simulink model "Demo_TrafficFlow_cs"  (Simulink 7.6)
settings: Solver=ode5  SolverMode=SingleTasking  StartTime=0.0  StopTime=60  FixedStep=1/20  RelTol=1e-3
PreLoadFcn:
  | check_NUTS_defined
  | try
  |     evalin('base', 'NUTS_defined;');
  | catch
  |     warningMsg = sprintf('PreScan Warning: \nUsing this model requires that MATLAB is configured for PreScan. The model may not display correctly.\n\n ==> Please open MATLAB with the dedicated buttons provided with PreScan');
  |     disp(warningMsg)
  |     warndlg(warningMsg,'WindowStyle','Modal');
  | end
  | 
  | model_closed = 0;
  | assignin('base',['model_closed_' bdroot],false);
InitFcn:
  | PreScanExperimentInitFcn(bdroot)
StopFcn:
  | PreScanExperimentStopFcn(bdroot)
undefined symbols (not defined in the file or in its folder): Advised_Speed_L1, Advised_Speed_L2, Advised_Speed_L3, DiscIntNeverNeededParam, spare_10, spare_5, spare_6, spare_7, spare_8, spare_9, State_L2, Throughput_L1, Throughput_L2, Throughput_L3, Velocity_L1, Velocity_L2, Velocity_L3
libraries: simulink x58, PreScanSimulinkLibrary x40, simulink_extras x32, PreScanUsefulBlocks x22, simulink_need_slupdate x3
(part 3 of 20)

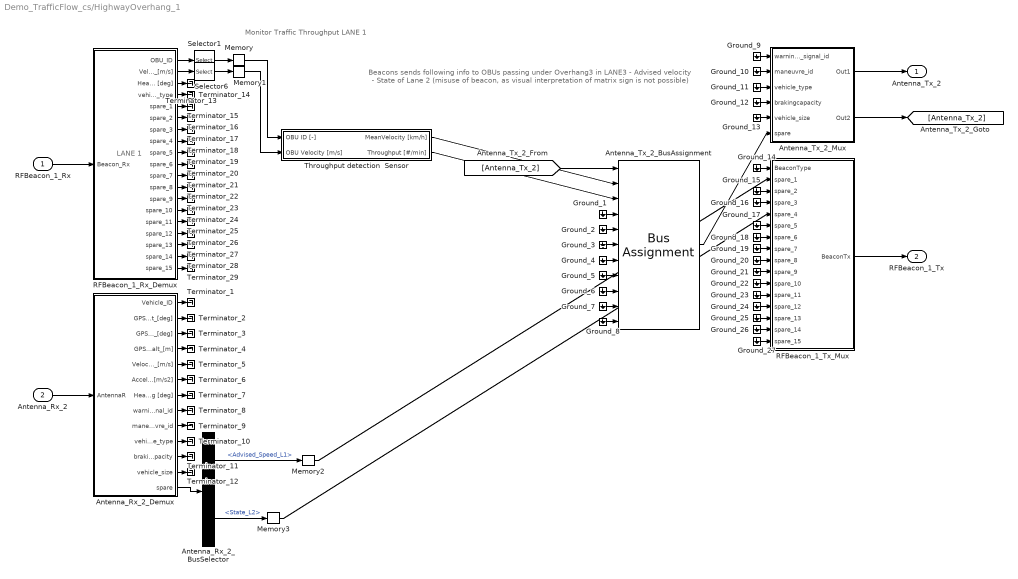
== system Demo_TrafficFlow_cs/HighwayOverhang_1 ==
  note: LANE 1
  note: Monitor Traffic Throughput LANE 1
  note: Beacons sends following info to OBUs
      | passing under Overhang3 in LANE3
      | - Advised velocity
      | - State of Lane 2 (misuse of beacon, as visual
      |    interpretation of matrix sign is not possible)
  RFBeacon_1_Rx : Inport  Tag=BeaconRxInport
  Antenna_Rx_2 : Inport  Tag=AntennaRInport  Port=2
  Antenna_Rx_2_BusSelector : BusSelector  Tag=BusAntennaR  OutputSignals=Advised_Speed_L1,State_L2
  Antenna_Rx_2_Demux : Subsystem  Tag=DemuxAntennaR  mask(PreScan Demux Block:){Channel=1}
      settings: DeleteFcn=PreScanBlockDeleteFcn;
      mask Display:
      | fprintf('Channel: %d',Channel)
      | port_label('output', 1, 'Vehicle_ID')
      | port_label('output', 2, 'GPS Pos Lat [deg]')
      | port_label('output', 3, 'GPS Pos Long [deg]')
      | port_label('output', 4, 'GPS Pos Alt [m]')
      | port_label('output', 5, 'Velocity [m/s]')
      | port_label('output', 6, 'Acceleration [m/s2]')
      | port_label('output', 7, 'Heading [deg]')
      | port_label('output', 8, 'Warning signal ID')
      | port_label('output', 9, 'Maneuvre ID')
      | port_label('output', 10, 'Vehicle Type')
      | port_label('output', 11, 'Braking Capacity')
      | port_label('output', 12, 'Vehicle Size')
      | port_label('output', 13, 'Spare')
  Antenna_Tx_2_BusAssignment : BusAssignment  Tag=BusAntennaT  AssignedSignals=Velocity_L1,Throughput_L1,Advised_Speed_L1,State_L2,spare_5,spare_6,spare_7,spare_8,spare_9,spare_10
  Antenna_Tx_2_Mux : Subsystem  Tag=MuxAntennaT  mask(PreScan Mux Block:){Channel=1, TimeStep=1/20}
      mask prompts: TimeStep "Time Step [s]"
      settings: DeleteFcn=PreScanBlockDeleteFcn;
      mask Display:
      | fprintf('Channel: %d',Channel)
      | port_label('input', 1, 'Warning signal ID')
      | port_label('input', 2, 'Maneuvre ID')
      | port_label('input', 3, 'Vehicle Type')
      | port_label('input', 4, 'Braking Capacity')
      | port_label('input', 5, 'Vehicle Size')
      | port_label('input', 6, 'spare')
  Ground_{1..27} (27 alike) : Ground
  Memory{,1..3} (4 alike) : Memory
  RFBeacon_1_Rx_Demux : Subsystem  Tag=DemuxAntennaR  mask(PreScan Demux Block:){Technology='RF'}
      mask init:
      | IconTxt = char('','','','','Technology:            ',['{\it',Technology,'}']);
      settings: DeleteFcn=PreScanBlockDeleteFcn;
      mask Display:
      | disp(IconTxt,'texmode','on')
      | port_label('output', 1, 'OBU ID [-]')
      | port_label('output', 2, 'OBU Velocity [m/s]')
      | port_label('output', 3, 'OBU Heading [deg]')
      | port_label('output', 4, 'Vehicle Type[-]')
      | port_label('output', 5, 'spare_1')
      | port_label('output', 6, 'spare_2')
      | port_label('output', 7, 'spare_3')
      | port_label('output', 8, 'spare_4')
      | port_label('output', 9, 'spare_5')
      | port_label('output', 10, 'spare_6')
      | port_label('output', 11, 'spare_7')
      | port_label('output', 12, 'spare_8')
      | port_label('output', 13, 'spare_9')
      | port_label('output', 14, 'spare_10')
      | port_label('output', 15, 'spare_11')
      | port_label('output', 16, 'spare_12')
      | port_label('output', 17, 'spare_13')
      | port_label('output', 18, 'spare_14')
      | port_label('output', 19, 'spare_15')
  RFBeacon_1_Tx_Mux : Subsystem  Tag=MuxBeaconTx  mask(PreScan Mux Block:){Technology='RF'}
      mask init:
      | IconTxt = char('','','','              Technology:',['{\it                ',Technology,'}']);
      settings: DeleteFcn=PreScanBlockDeleteFcn;
      mask Display:
      | disp(IconTxt,'texmode','on')
      | port_label('input', 1, 'Beacon Type [-]')
      | port_label('input', 2, 'Advised_Velocity')
      | port_label('input', 3, 'CarID_Lane1')
      | port_label('input', 4, 'CarID_Lane3')
      | port_label('input', 5, 'State_Lane2')
      | port_label('input', 6, 'spare_5')
      | port_label('input', 7, 'spare_6')
      | port_label('input', 8, 'spare_7')
      | port_label('input', 9, 'spare_8')
      | port_label('input', 10, 'spare_9')
      | port_label('input', 11, 'spare_10')
      | port_label('input', 12, 'spare_11')
      | port_label('input', 13, 'spare_12')
      | port_label('input', 14, 'spare_13')
      | port_label('input', 15, 'spare_14')
      | port_label('input', 16, 'spare_15')
  Selector1 : Selector  InputPortWidth=5  IndexOptions=Index vector (dialog)  Indices=[1]  OutputSizes=1
  Selector6 : Selector  InputPortWidth=5  IndexOptions=Index vector (dialog)  Indices=[1]  OutputSizes=1
  Terminator_{1..29} (29 alike) : Terminator
  Throughput detection  Sensor : Subsystem
  Antenna_Tx_2 : Outport  Tag=AntennaTOutport
  RFBeacon_1_Tx : Outport  Tag=AntennaTOutport  Port=2
  wires:
  Selector6/1 -> Memory1/1
  Selector1/1 -> Memory/1
  Memory/1 -> Throughput detection  Sensor/1(OBU ID [-])
  Memory1/1 -> Throughput detection  Sensor/2(OBU Velocity [m/s])
  RFBeacon_1_Rx/1 -> RFBeacon_1_Rx_Demux/1(Beacon_Rx)
  Antenna_Rx_2_Demux/13(spare) -> Antenna_Rx_2_BusSelector/1
  Antenna_Rx_2/1 -> Antenna_Rx_2_Demux/1(AntennaR)
  Antenna_Tx_2_Mux/2(Out2) -> [Antenna_Tx_2]
  [Antenna_Tx_2] -> Antenna_Tx_2_BusAssignment/1
  Antenna_Tx_2_BusAssignment/1 -> Antenna_Tx_2_Mux/6(spare)
  Antenna_Tx_2_Mux/1(Out1) -> Antenna_Tx_2/1
  RFBeacon_1_Tx_Mux/1(BeaconTx) -> RFBeacon_1_Tx/1
  Memory3/1 -> RFBeacon_1_Tx_Mux/5(spare_4)
  RFBeacon_1_Rx_Demux/2(Velocity_[m/s]) -> Selector6/1
  RFBeacon_1_Rx_Demux/1(OBU_ID) -> Selector1/1
  Throughput detection  Sensor/2(Throughput [#/min]) -> Antenna_Tx_2_BusAssignment/3
  Throughput detection  Sensor/1(MeanVelocity [km/h]) -> Antenna_Tx_2_BusAssignment/2
  Memory2/1 -> RFBeacon_1_Tx_Mux/2(spare_1)
  Antenna_Rx_2_BusSelector/1 -> Memory2/1  "<Advised_Speed_L1>"
  Antenna_Rx_2_BusSelector/2 -> Memory3/1  "<State_L2>"
  Antenna_Rx_2_Demux/1(Vehicle_ID) -> Terminator_1/1
  Antenna_Rx_2_Demux/2(GPS_pos_lat_[deg]) -> Terminator_2/1
  Antenna_Rx_2_Demux/3(GPS_pos_long_[deg]) -> Terminator_3/1
  Antenna_Rx_2_Demux/4(GPS_pos_alt_[m]) -> Terminator_4/1
  Antenna_Rx_2_Demux/5(Velocity_[m/s]) -> Terminator_5/1
  Antenna_Rx_2_Demux/6(Acceleration_[m/s2]) -> Terminator_6/1
  Antenna_Rx_2_Demux/7(Heading [deg]) -> Terminator_7/1
  Antenna_Rx_2_Demux/8(warning_signal_id) -> Terminator_8/1
  Antenna_Rx_2_Demux/9(maneuvre_id) -> Terminator_9/1
  Antenna_Rx_2_Demux/10(vehicle_type) -> Terminator_10/1
  Antenna_Rx_2_Demux/11(brakingcapacity) -> Terminator_11/1
  Antenna_Rx_2_Demux/12(vehicle_size) -> Terminator_12/1
  Ground_1/1 -> Antenna_Tx_2_BusAssignment/4
  Ground_2/1 -> Antenna_Tx_2_BusAssignment/5
  Ground_3/1 -> Antenna_Tx_2_BusAssignment/6
  Ground_4/1 -> Antenna_Tx_2_BusAssignment/7
  Ground_5/1 -> Antenna_Tx_2_BusAssignment/8
  Ground_6/1 -> Antenna_Tx_2_BusAssignment/9
  Ground_7/1 -> Antenna_Tx_2_BusAssignment/10
  Ground_8/1 -> Antenna_Tx_2_BusAssignment/11
  Ground_9/1 -> Antenna_Tx_2_Mux/1(warning_signal_id)
  Ground_10/1 -> Antenna_Tx_2_Mux/2(maneuvre_id)
  Ground_11/1 -> Antenna_Tx_2_Mux/3(vehicle_type)
  Ground_12/1 -> Antenna_Tx_2_Mux/4(brakingcapacity)
  Ground_13/1 -> Antenna_Tx_2_Mux/5(vehicle_size)
  RFBeacon_1_Rx_Demux/3(Heading [deg]) -> Terminator_13/1
  RFBeacon_1_Rx_Demux/4(vehicle_type) -> Terminator_14/1
  RFBeacon_1_Rx_Demux/5(spare_1) -> Terminator_15/1
  RFBeacon_1_Rx_Demux/6(spare_2) -> Terminator_16/1
  RFBeacon_1_Rx_Demux/7(spare_3) -> Terminator_17/1
  RFBeacon_1_Rx_Demux/8(spare_4) -> Terminator_18/1
  RFBeacon_1_Rx_Demux/9(spare_5) -> Terminator_19/1
  RFBeacon_1_Rx_Demux/10(spare_6) -> Terminator_20/1
  RFBeacon_1_Rx_Demux/11(spare_7) -> Terminator_21/1
  RFBeacon_1_Rx_Demux/12(spare_8) -> Terminator_22/1
  RFBeacon_1_Rx_Demux/13(spare_9) -> Terminator_23/1
  RFBeacon_1_Rx_Demux/14(spare_10) -> Terminator_24/1
  RFBeacon_1_Rx_Demux/15(spare_11) -> Terminator_25/1
  RFBeacon_1_Rx_Demux/16(spare_12) -> Terminator_26/1
  RFBeacon_1_Rx_Demux/17(spare_13) -> Terminator_27/1
  RFBeacon_1_Rx_Demux/18(spare_14) -> Terminator_28/1
  RFBeacon_1_Rx_Demux/19(spare_15) -> Terminator_29/1
  Ground_14/1 -> RFBeacon_1_Tx_Mux/1(BeaconType)
  Ground_15/1 -> RFBeacon_1_Tx_Mux/3(spare_2)
  Ground_16/1 -> RFBeacon_1_Tx_Mux/4(spare_3)
  Ground_17/1 -> RFBeacon_1_Tx_Mux/6(spare_5)
  Ground_18/1 -> RFBeacon_1_Tx_Mux/7(spare_6)
  Ground_19/1 -> RFBeacon_1_Tx_Mux/8(spare_7)
  Ground_20/1 -> RFBeacon_1_Tx_Mux/9(spare_8)
  Ground_21/1 -> RFBeacon_1_Tx_Mux/10(spare_9)
  Ground_22/1 -> RFBeacon_1_Tx_Mux/11(spare_10)
  Ground_23/1 -> RFBeacon_1_Tx_Mux/12(spare_11)
  Ground_24/1 -> RFBeacon_1_Tx_Mux/13(spare_12)
  Ground_25/1 -> RFBeacon_1_Tx_Mux/14(spare_13)
  Ground_26/1 -> RFBeacon_1_Tx_Mux/15(spare_14)
  Ground_27/1 -> RFBeacon_1_Tx_Mux/16(spare_15)

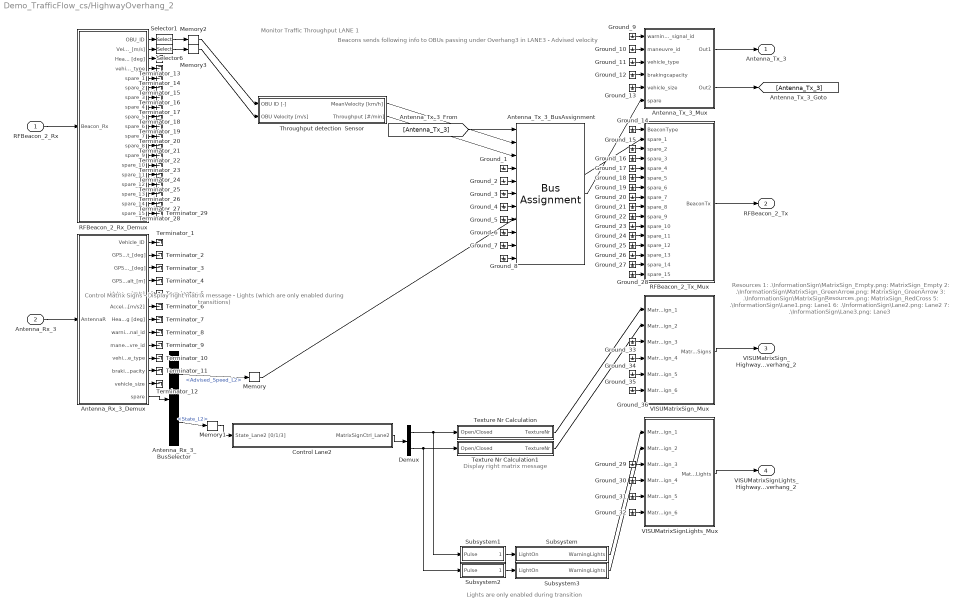
== system Demo_TrafficFlow_cs/HighwayOverhang_2 ==
  note: Lights are only enabled during transition
  note: Display right matrix message
  note: Monitor Traffic Throughput LANE 1
  note: Beacons sends following info to OBUs
      | passing under Overhang3 in LANE3
      | - Advised velocity
  note: Control Matrix Signs
      | - Display right matrix message
      | - Lights (which are only enabled during transitions)
  note: Resources
      |
      | 1: .\InformationSign\MatrixSign_Empty.png: MatrixSign_Empty
      | 2: .\InformationSign\MatrixSign_GreenArrow.png: MatrixSign_GreenArrow
      | 3: .\InformationSign\MatrixSign_RedCross.png: MatrixSign_RedCross
      | 5: .\InformationSign\Lane1.png: Lane1
      | 6: .\InformationSign\Lane2.png: Lane2
      | 7: .\InformationSign\Lane3.png: Lane3
  note: Resources
  RFBeacon_2_Rx : Inport  Tag=BeaconRxInport
  Antenna_Rx_3 : Inport  Tag=AntennaRInport  Port=2
  Antenna_Rx_3_BusSelector : BusSelector  Tag=BusAntennaR  OutputSignals=Advised_Speed_L2,State_L2
  Antenna_Rx_3_Demux : Subsystem  Tag=DemuxAntennaR  mask(PreScan Demux Block:){Channel=2}
      settings: DeleteFcn=PreScanBlockDeleteFcn;
      mask Display:
      | fprintf('Channel: %d',Channel)
      | port_label('output', 1, 'Vehicle_ID')
      | port_label('output', 2, 'GPS Pos Lat [deg]')
      | port_label('output', 3, 'GPS Pos Long [deg]')
      | port_label('output', 4, 'GPS Pos Alt [m]')
      | port_label('output', 5, 'Velocity [m/s]')
      | port_label('output', 6, 'Acceleration [m/s2]')
      | port_label('output', 7, 'Heading [deg]')
      | port_label('output', 8, 'Warning signal ID')
      | port_label('output', 9, 'Maneuvre ID')
      | port_label('output', 10, 'Vehicle Type')
      | port_label('output', 11, 'Braking Capacity')
      | port_label('output', 12, 'Vehicle Size')
      | port_label('output', 13, 'Spare')
  Antenna_Tx_3_BusAssignment : BusAssignment  Tag=BusAntennaT  AssignedSignals=Velocity_L2,Throughput_L2,Advised_Speed_L2,State_L2,spare_5,spare_6,spare_7,spare_8,spare_9,spare_10
  Antenna_Tx_3_Mux : Subsystem  Tag=MuxAntennaT  mask(PreScan Mux Block:){Channel=2, TimeStep=1/20}
      mask prompts: TimeStep "Time Step [s]"
      settings: DeleteFcn=PreScanBlockDeleteFcn;
      mask Display:
      | fprintf('Channel: %d',Channel)
      | port_label('input', 1, 'Warning signal ID')
      | port_label('input', 2, 'Maneuvre ID')
      | port_label('input', 3, 'Vehicle Type')
      | port_label('input', 4, 'Braking Capacity')
      | port_label('input', 5, 'Vehicle Size')
      | port_label('input', 6, 'spare')
  Control Lane2 : Subsystem
  Demux : Demux  Outputs=2
  Ground_{1..36} (36 alike) : Ground
  Memory{,1..3} (4 alike) : Memory
  RFBeacon_2_Rx_Demux : Subsystem  Tag=DemuxAntennaR  mask(PreScan Demux Block:){Technology='RF'}
      mask init:
      | IconTxt = char('','','','','Technology:            ',['{\it',Technology,'}']);
      settings: DeleteFcn=PreScanBlockDeleteFcn;
      mask Display:
      | disp(IconTxt,'texmode','on')
      | port_label('output', 1, 'OBU ID [-]')
      | port_label('output', 2, 'OBU Velocity [m/s]')
      | port_label('output', 3, 'OBU Heading [deg]')
      | port_label('output', 4, 'Vehicle Type[-]')
      | port_label('output', 5, 'spare_1')
      | port_label('output', 6, 'spare_2')
      | port_label('output', 7, 'spare_3')
      | port_label('output', 8, 'spare_4')
      | port_label('output', 9, 'spare_5')
      | port_label('output', 10, 'spare_6')
      | port_label('output', 11, 'spare_7')
      | port_label('output', 12, 'spare_8')
      | port_label('output', 13, 'spare_9')
      | port_label('output', 14, 'spare_10')
      | port_label('output', 15, 'spare_11')
      | port_label('output', 16, 'spare_12')
      | port_label('output', 17, 'spare_13')
      | port_label('output', 18, 'spare_14')
      | port_label('output', 19, 'spare_15')
  RFBeacon_2_Tx_Mux : Subsystem  Tag=MuxBeaconTx  mask(PreScan Mux Block:){Technology='RF'}
      mask init:
      | IconTxt = char('','','','              Technology:',['{\it                ',Technology,'}']);
      settings: DeleteFcn=PreScanBlockDeleteFcn;
      mask Display:
      | disp(IconTxt,'texmode','on')
      | port_label('input', 1, 'Beacon Type [-]')
      | port_label('input', 2, 'Advised_Velocity')
      | port_label('input', 3, 'CarID_Lane1')
      | port_label('input', 4, 'CarID_Lane3')
      | port_label('input', 5, 'State_Lane2')
      | port_label('input', 6, 'spare_5')
      | port_label('input', 7, 'spare_6')
      | port_label('input', 8, 'spare_7')
      | port_label('input', 9, 'spare_8')
      | port_label('input', 10, 'spare_9')
      | port_label('input', 11, 'spare_10')
      | port_label('input', 12, 'spare_11')
      | port_label('input', 13, 'spare_12')
      | port_label('input', 14, 'spare_13')
      | port_label('input', 15, 'spare_14')
      | port_label('input', 16, 'spare_15')
  Selector1 : Selector  InputPortWidth=5  IndexOptions=Index vector (dialog)  Indices=[1]  OutputSizes=1
  Selector6 : Selector  InputPortWidth=5  IndexOptions=Index vector (dialog)  Indices=[1]  OutputSizes=1
  Subsystem : Subsystem
  Subsystem1 : Subsystem  mask{t_light_on=10}
      mask prompts: t_light_on "Set duration of warning lights activation time"
  Subsystem2 : Subsystem  mask{t_light_on=3}
      mask prompts: t_light_on "Set duration of warning lights activation time"
  Subsystem3 : Subsystem
  Terminator_{1..29} (29 alike) : Terminator
  Texture Nr Calculation : Subsystem
  Texture Nr Calculation1 : Subsystem
  Throughput detection  Sensor : Subsystem
  VISUMatrixSignLights_Mux : Subsystem  Tag=MuxMatrixSignLights
      settings: DeleteFcn=PreScanBlockDeleteFcn;
      mask Type: PreScan Mux Block:
  VISUMatrixSign_Mux : Subsystem  Tag=MuxMatrixSign
      settings: DeleteFcn=PreScanBlockDeleteFcn;
      mask Type: PreScan Mux Block:
  Antenna_Tx_3 : Outport  Tag=AntennaTOutport
  RFBeacon_2_Tx : Outport  Tag=AntennaTOutport  Port=2
  VISUMatrixSign_HighwayOverhang_2 : Outport  Tag=VisuMatrixSignOutport  Port=3  SampleTime=1/20
  VISUMatrixSignLights_HighwayOverhang_2 : Outport  Tag=VisuMatrixSignLightsOutport  Port=4  SampleTime=1/20
  wires:
  Demux/1 -> Texture Nr Calculation/1(Open/Closed), Subsystem1/1(Pulse)
  Demux/2 -> Texture Nr Calculation1/1(Open/Closed), Subsystem2/1(Pulse)
  Subsystem2/1(LightOn) -> Subsystem3/1(LightOn)
  Subsystem1/1(LightOn) -> Subsystem/1(LightOn)
  Selector1/1 -> Memory2/1
  Selector6/1 -> Memory3/1
  Control Lane2/1(MatrixSignCtrl_Lane2) -> Demux/1
  Memory1/1 -> Control Lane2/1(State_Lane2 [0/1/3])
  Memory2/1 -> Throughput detection  Sensor/1(OBU ID [-])
  Memory3/1 -> Throughput detection  Sensor/2(OBU Velocity [m/s])
  RFBeacon_2_Rx/1 -> RFBeacon_2_Rx_Demux/1(Beacon_Rx)
  Antenna_Rx_3_Demux/13(spare) -> Antenna_Rx_3_BusSelector/1
  Antenna_Rx_3/1 -> Antenna_Rx_3_Demux/1(AntennaR)
  Antenna_Tx_3_Mux/2(Out2) -> [Antenna_Tx_3]
  [Antenna_Tx_3] -> Antenna_Tx_3_BusAssignment/1
  Antenna_Tx_3_BusAssignment/1 -> Antenna_Tx_3_Mux/6(spare)
  Antenna_Tx_3_Mux/1(Out1) -> Antenna_Tx_3/1
  RFBeacon_2_Tx_Mux/1(BeaconTx) -> RFBeacon_2_Tx/1
  VISUMatrixSign_Mux/1(MatrixSigns) -> VISUMatrixSign_HighwayOverhang_2/1
  VISUMatrixSignLights_Mux/1(MatrixSignLights) -> VISUMatrixSignLights_HighwayOverhang_2/1
  RFBeacon_2_Rx_Demux/1(OBU_ID) -> Selector1/1
  RFBeacon_2_Rx_Demux/2(Velocity_[m/s]) -> Selector6/1
  Subsystem/1(WarningLights) -> VISUMatrixSignLights_Mux/1(MatrixSign_1)
  Subsystem3/1(WarningLights) -> VISUMatrixSignLights_Mux/2(MatrixSign_2)
  Texture Nr Calculation/1(TextureNr) -> VISUMatrixSign_Mux/1(MatrixSign_1)
  Texture Nr Calculation1/1(TextureNr) -> VISUMatrixSign_Mux/2(MatrixSign_2)
  Memory/1 -> RFBeacon_2_Tx_Mux/2(spare_1)
  Throughput detection  Sensor/1(MeanVelocity [km/h]) -> Antenna_Tx_3_BusAssignment/2
  Throughput detection  Sensor/2(Throughput [#/min]) -> Antenna_Tx_3_BusAssignment/3
  Antenna_Rx_3_BusSelector/1 -> Memory/1  "<Advised_Speed_L2>"
  Antenna_Rx_3_BusSelector/2 -> Memory1/1  "<State_L2>"
  Antenna_Rx_3_Demux/1(Vehicle_ID) -> Terminator_1/1
  Antenna_Rx_3_Demux/2(GPS_pos_lat_[deg]) -> Terminator_2/1
  Antenna_Rx_3_Demux/3(GPS_pos_long_[deg]) -> Terminator_3/1
  Antenna_Rx_3_Demux/4(GPS_pos_alt_[m]) -> Terminator_4/1
  Antenna_Rx_3_Demux/5(Velocity_[m/s]) -> Terminator_5/1
  Antenna_Rx_3_Demux/6(Acceleration_[m/s2]) -> Terminator_6/1
  Antenna_Rx_3_Demux/7(Heading [deg]) -> Terminator_7/1
  Antenna_Rx_3_Demux/8(warning_signal_id) -> Terminator_8/1
  Antenna_Rx_3_Demux/9(maneuvre_id) -> Terminator_9/1
  Antenna_Rx_3_Demux/10(vehicle_type) -> Terminator_10/1
  Antenna_Rx_3_Demux/11(brakingcapacity) -> Terminator_11/1
  Antenna_Rx_3_Demux/12(vehicle_size) -> Terminator_12/1
  Ground_1/1 -> Antenna_Tx_3_BusAssignment/4
  Ground_2/1 -> Antenna_Tx_3_BusAssignment/5
  Ground_3/1 -> Antenna_Tx_3_BusAssignment/6
  Ground_4/1 -> Antenna_Tx_3_BusAssignment/7
  Ground_5/1 -> Antenna_Tx_3_BusAssignment/8
  Ground_6/1 -> Antenna_Tx_3_BusAssignment/9
  Ground_7/1 -> Antenna_Tx_3_BusAssignment/10
  Ground_8/1 -> Antenna_Tx_3_BusAssignment/11
  Ground_9/1 -> Antenna_Tx_3_Mux/1(warning_signal_id)
  Ground_10/1 -> Antenna_Tx_3_Mux/2(maneuvre_id)
  Ground_11/1 -> Antenna_Tx_3_Mux/3(vehicle_type)
  Ground_12/1 -> Antenna_Tx_3_Mux/4(brakingcapacity)
  Ground_13/1 -> Antenna_Tx_3_Mux/5(vehicle_size)
  RFBeacon_2_Rx_Demux/3(Heading [deg]) -> Terminator_13/1
  RFBeacon_2_Rx_Demux/4(vehicle_type) -> Terminator_14/1
  RFBeacon_2_Rx_Demux/5(spare_1) -> Terminator_15/1
  RFBeacon_2_Rx_Demux/6(spare_2) -> Terminator_16/1
  RFBeacon_2_Rx_Demux/7(spare_3) -> Terminator_17/1
  RFBeacon_2_Rx_Demux/8(spare_4) -> Terminator_18/1
  RFBeacon_2_Rx_Demux/9(spare_5) -> Terminator_19/1
  RFBeacon_2_Rx_Demux/10(spare_6) -> Terminator_20/1
  RFBeacon_2_Rx_Demux/11(spare_7) -> Terminator_21/1
  RFBeacon_2_Rx_Demux/12(spare_8) -> Terminator_22/1
  RFBeacon_2_Rx_Demux/13(spare_9) -> Terminator_23/1
  RFBeacon_2_Rx_Demux/14(spare_10) -> Terminator_24/1
  RFBeacon_2_Rx_Demux/15(spare_11) -> Terminator_25/1
  RFBeacon_2_Rx_Demux/16(spare_12) -> Terminator_26/1
  RFBeacon_2_Rx_Demux/17(spare_13) -> Terminator_27/1
  RFBeacon_2_Rx_Demux/18(spare_14) -> Terminator_28/1
  RFBeacon_2_Rx_Demux/19(spare_15) -> Terminator_29/1
  Ground_14/1 -> RFBeacon_2_Tx_Mux/1(BeaconType)
  Ground_15/1 -> RFBeacon_2_Tx_Mux/3(spare_2)
  Ground_16/1 -> RFBeacon_2_Tx_Mux/4(spare_3)
  Ground_17/1 -> RFBeacon_2_Tx_Mux/5(spare_4)
  Ground_18/1 -> RFBeacon_2_Tx_Mux/6(spare_5)
  Ground_19/1 -> RFBeacon_2_Tx_Mux/7(spare_6)
  Ground_20/1 -> RFBeacon_2_Tx_Mux/8(spare_7)
  Ground_21/1 -> RFBeacon_2_Tx_Mux/9(spare_8)
  Ground_22/1 -> RFBeacon_2_Tx_Mux/10(spare_9)
  Ground_23/1 -> RFBeacon_2_Tx_Mux/11(spare_10)
  Ground_24/1 -> RFBeacon_2_Tx_Mux/12(spare_11)
  Ground_25/1 -> RFBeacon_2_Tx_Mux/13(spare_12)
  Ground_26/1 -> RFBeacon_2_Tx_Mux/14(spare_13)
  Ground_27/1 -> RFBeacon_2_Tx_Mux/15(spare_14)
  Ground_28/1 -> RFBeacon_2_Tx_Mux/16(spare_15)
  Ground_29/1 -> VISUMatrixSignLights_Mux/3(MatrixSign_3)
  Ground_30/1 -> VISUMatrixSignLights_Mux/4(MatrixSign_4)
  Ground_31/1 -> VISUMatrixSignLights_Mux/5(MatrixSign_5)
  Ground_32/1 -> VISUMatrixSignLights_Mux/6(MatrixSign_6)
  Ground_33/1 -> VISUMatrixSign_Mux/3(MatrixSign_3)
  Ground_34/1 -> VISUMatrixSign_Mux/4(MatrixSign_4)
  Ground_35/1 -> VISUMatrixSign_Mux/5(MatrixSign_5)
  Ground_36/1 -> VISUMatrixSign_Mux/6(MatrixSign_6)

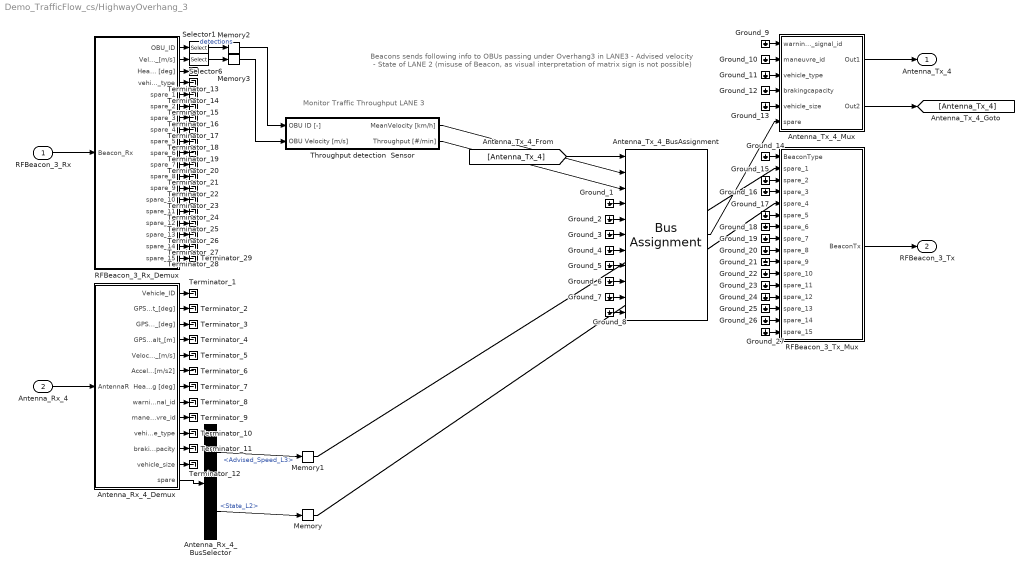
== system Demo_TrafficFlow_cs/HighwayOverhang_3 ==
  note: Monitor Traffic Throughput LANE 3
  note: Beacons sends following info to OBUs
      | passing under Overhang3 in LANE3
      | - Advised velocity
      | - State of LANE 2 (misuse of Beacon, as visual
      |    interpretation of matrix sign is not possible)
  RFBeacon_3_Rx : Inport  Tag=BeaconRxInport
  Antenna_Rx_4 : Inport  Tag=AntennaRInport  Port=2
  Antenna_Rx_4_BusSelector : BusSelector  Tag=BusAntennaR  OutputSignals=Advised_Speed_L3,State_L2
  Antenna_Rx_4_Demux : Subsystem  Tag=DemuxAntennaR  mask(PreScan Demux Block:){Channel=3}
      settings: DeleteFcn=PreScanBlockDeleteFcn;
      mask Display:
      | fprintf('Channel: %d',Channel)
      | port_label('output', 1, 'Vehicle_ID')
      | port_label('output', 2, 'GPS Pos Lat [deg]')
      | port_label('output', 3, 'GPS Pos Long [deg]')
      | port_label('output', 4, 'GPS Pos Alt [m]')
      | port_label('output', 5, 'Velocity [m/s]')
      | port_label('output', 6, 'Acceleration [m/s2]')
      | port_label('output', 7, 'Heading [deg]')
      | port_label('output', 8, 'Warning signal ID')
      | port_label('output', 9, 'Maneuvre ID')
      | port_label('output', 10, 'Vehicle Type')
      | port_label('output', 11, 'Braking Capacity')
      | port_label('output', 12, 'Vehicle Size')
      | port_label('output', 13, 'Spare')
  Antenna_Tx_4_BusAssignment : BusAssignment  Tag=BusAntennaT  AssignedSignals=Velocity_L3,Throughput_L3,Advised_Speed_L3,State_L2,spare_5,spare_6,spare_7,spare_8,spare_9,spare_10
  Antenna_Tx_4_Mux : Subsystem  Tag=MuxAntennaT  mask(PreScan Mux Block:){Channel=3, TimeStep=1/20}
      mask prompts: TimeStep "Time Step [s]"
      settings: DeleteFcn=PreScanBlockDeleteFcn;
      mask Display:
      | fprintf('Channel: %d',Channel)
      | port_label('input', 1, 'Warning signal ID')
      | port_label('input', 2, 'Maneuvre ID')
      | port_label('input', 3, 'Vehicle Type')
      | port_label('input', 4, 'Braking Capacity')
      | port_label('input', 5, 'Vehicle Size')
      | port_label('input', 6, 'spare')
  Ground_{1..27} (27 alike) : Ground
  Memory{,1..3} (4 alike) : Memory
  RFBeacon_3_Rx_Demux : Subsystem  Tag=DemuxAntennaR  mask(PreScan Demux Block:){Technology='RF'}
      mask init:
      | IconTxt = char('','','','','Technology:            ',['{\it',Technology,'}']);
      settings: DeleteFcn=PreScanBlockDeleteFcn;
      mask Display:
      | disp(IconTxt,'texmode','on')
      | port_label('output', 1, 'OBU ID [-]')
      | port_label('output', 2, 'OBU Velocity [m/s]')
      | port_label('output', 3, 'OBU Heading [deg]')
      | port_label('output', 4, 'Vehicle Type[-]')
      | port_label('output', 5, 'spare_1')
      | port_label('output', 6, 'spare_2')
      | port_label('output', 7, 'spare_3')
      | port_label('output', 8, 'spare_4')
      | port_label('output', 9, 'spare_5')
      | port_label('output', 10, 'spare_6')
      | port_label('output', 11, 'spare_7')
      | port_label('output', 12, 'spare_8')
      | port_label('output', 13, 'spare_9')
      | port_label('output', 14, 'spare_10')
      | port_label('output', 15, 'spare_11')
      | port_label('output', 16, 'spare_12')
      | port_label('output', 17, 'spare_13')
      | port_label('output', 18, 'spare_14')
      | port_label('output', 19, 'spare_15')
  RFBeacon_3_Tx_Mux : Subsystem  Tag=MuxBeaconTx  mask(PreScan Mux Block:){Technology='RF'}
      mask init:
      | IconTxt = char('','','','              Technology:',['{\it                ',Technology,'}']);
      settings: DeleteFcn=PreScanBlockDeleteFcn;
      mask Display:
      | disp(IconTxt,'texmode','on')
      | port_label('input', 1, 'Beacon Type [-]')
      | port_label('input', 2, 'Advised_Velocity')
      | port_label('input', 3, 'CarID_Lane1')
      | port_label('input', 4, 'CarID_Lane3')
      | port_label('input', 5, 'State_Lane2')
      | port_label('input', 6, 'spare_5')
      | port_label('input', 7, 'spare_6')
      | port_label('input', 8, 'spare_7')
      | port_label('input', 9, 'spare_8')
      | port_label('input', 10, 'spare_9')
      | port_label('input', 11, 'spare_10')
      | port_label('input', 12, 'spare_11')
      | port_label('input', 13, 'spare_12')
      | port_label('input', 14, 'spare_13')
      | port_label('input', 15, 'spare_14')
      | port_label('input', 16, 'spare_15')
  Selector1 : Selector  InputPortWidth=5  IndexOptions=Index vector (dialog)  Indices=[1]  OutputSizes=1
  Selector6 : Selector  InputPortWidth=5  IndexOptions=Index vector (dialog)  Indices=[1]  OutputSizes=1
  Terminator_{1..29} (29 alike) : Terminator
  Throughput detection  Sensor : Subsystem
  Antenna_Tx_4 : Outport  Tag=AntennaTOutport
  RFBeacon_3_Tx : Outport  Tag=AntennaTOutport  Port=2
  wires:
  Selector1/1 -> Memory2/1  "detections"
  Selector6/1 -> Memory3/1
  Memory2/1 -> Throughput detection  Sensor/1(OBU ID [-])
  Memory3/1 -> Throughput detection  Sensor/2(OBU Velocity [m/s])
  RFBeacon_3_Rx/1 -> RFBeacon_3_Rx_Demux/1(Beacon_Rx)
  Antenna_Rx_4_Demux/13(spare) -> Antenna_Rx_4_BusSelector/1
  Antenna_Rx_4/1 -> Antenna_Rx_4_Demux/1(AntennaR)
  Antenna_Tx_4_Mux/2(Out2) -> [Antenna_Tx_4]
  [Antenna_Tx_4] -> Antenna_Tx_4_BusAssignment/1
  Antenna_Tx_4_BusAssignment/1 -> Antenna_Tx_4_Mux/6(spare)
  Antenna_Tx_4_Mux/1(Out1) -> Antenna_Tx_4/1
  RFBeacon_3_Tx_Mux/1(BeaconTx) -> RFBeacon_3_Tx/1
  RFBeacon_3_Rx_Demux/1(OBU_ID) -> Selector1/1
  RFBeacon_3_Rx_Demux/2(Velocity_[m/s]) -> Selector6/1
  Memory/1 -> RFBeacon_3_Tx_Mux/5(spare_4)
  Memory1/1 -> RFBeacon_3_Tx_Mux/2(spare_1)
  Throughput detection  Sensor/1(MeanVelocity [km/h]) -> Antenna_Tx_4_BusAssignment/2
  Throughput detection  Sensor/2(Throughput [#/min]) -> Antenna_Tx_4_BusAssignment/3
  Antenna_Rx_4_BusSelector/1 -> Memory1/1  "<Advised_Speed_L3>"
  Antenna_Rx_4_BusSelector/2 -> Memory/1  "<State_L2>"
  Antenna_Rx_4_Demux/1(Vehicle_ID) -> Terminator_1/1
  Antenna_Rx_4_Demux/2(GPS_pos_lat_[deg]) -> Terminator_2/1
  Antenna_Rx_4_Demux/3(GPS_pos_long_[deg]) -> Terminator_3/1
  Antenna_Rx_4_Demux/4(GPS_pos_alt_[m]) -> Terminator_4/1
  Antenna_Rx_4_Demux/5(Velocity_[m/s]) -> Terminator_5/1
  Antenna_Rx_4_Demux/6(Acceleration_[m/s2]) -> Terminator_6/1
  Antenna_Rx_4_Demux/7(Heading [deg]) -> Terminator_7/1
  Antenna_Rx_4_Demux/8(warning_signal_id) -> Terminator_8/1
  Antenna_Rx_4_Demux/9(maneuvre_id) -> Terminator_9/1
  Antenna_Rx_4_Demux/10(vehicle_type) -> Terminator_10/1
  Antenna_Rx_4_Demux/11(brakingcapacity) -> Terminator_11/1
  Antenna_Rx_4_Demux/12(vehicle_size) -> Terminator_12/1
  Ground_1/1 -> Antenna_Tx_4_BusAssignment/4
  Ground_2/1 -> Antenna_Tx_4_BusAssignment/5
  Ground_3/1 -> Antenna_Tx_4_BusAssignment/6
  Ground_4/1 -> Antenna_Tx_4_BusAssignment/7
  Ground_5/1 -> Antenna_Tx_4_BusAssignment/8
  Ground_6/1 -> Antenna_Tx_4_BusAssignment/9
  Ground_7/1 -> Antenna_Tx_4_BusAssignment/10
  Ground_8/1 -> Antenna_Tx_4_BusAssignment/11
  Ground_9/1 -> Antenna_Tx_4_Mux/1(warning_signal_id)
  Ground_10/1 -> Antenna_Tx_4_Mux/2(maneuvre_id)
  Ground_11/1 -> Antenna_Tx_4_Mux/3(vehicle_type)
  Ground_12/1 -> Antenna_Tx_4_Mux/4(brakingcapacity)
  Ground_13/1 -> Antenna_Tx_4_Mux/5(vehicle_size)
  RFBeacon_3_Rx_Demux/3(Heading [deg]) -> Terminator_13/1
  RFBeacon_3_Rx_Demux/4(vehicle_type) -> Terminator_14/1
  RFBeacon_3_Rx_Demux/5(spare_1) -> Terminator_15/1
  RFBeacon_3_Rx_Demux/6(spare_2) -> Terminator_16/1
  RFBeacon_3_Rx_Demux/7(spare_3) -> Terminator_17/1
  RFBeacon_3_Rx_Demux/8(spare_4) -> Terminator_18/1
  RFBeacon_3_Rx_Demux/9(spare_5) -> Terminator_19/1
  RFBeacon_3_Rx_Demux/10(spare_6) -> Terminator_20/1
  RFBeacon_3_Rx_Demux/11(spare_7) -> Terminator_21/1
  RFBeacon_3_Rx_Demux/12(spare_8) -> Terminator_22/1
  RFBeacon_3_Rx_Demux/13(spare_9) -> Terminator_23/1
  RFBeacon_3_Rx_Demux/14(spare_10) -> Terminator_24/1
  RFBeacon_3_Rx_Demux/15(spare_11) -> Terminator_25/1
  RFBeacon_3_Rx_Demux/16(spare_12) -> Terminator_26/1
  RFBeacon_3_Rx_Demux/17(spare_13) -> Terminator_27/1
  RFBeacon_3_Rx_Demux/18(spare_14) -> Terminator_28/1
  RFBeacon_3_Rx_Demux/19(spare_15) -> Terminator_29/1
  Ground_14/1 -> RFBeacon_3_Tx_Mux/1(BeaconType)
  Ground_15/1 -> RFBeacon_3_Tx_Mux/3(spare_2)
  Ground_16/1 -> RFBeacon_3_Tx_Mux/4(spare_3)
  Ground_17/1 -> RFBeacon_3_Tx_Mux/6(spare_5)
  Ground_18/1 -> RFBeacon_3_Tx_Mux/7(spare_6)
  Ground_19/1 -> RFBeacon_3_Tx_Mux/8(spare_7)
  Ground_20/1 -> RFBeacon_3_Tx_Mux/9(spare_8)
  Ground_21/1 -> RFBeacon_3_Tx_Mux/10(spare_9)
  Ground_22/1 -> RFBeacon_3_Tx_Mux/11(spare_10)
  Ground_23/1 -> RFBeacon_3_Tx_Mux/12(spare_11)
  Ground_24/1 -> RFBeacon_3_Tx_Mux/13(spare_12)
  Ground_25/1 -> RFBeacon_3_Tx_Mux/14(spare_13)
  Ground_26/1 -> RFBeacon_3_Tx_Mux/15(spare_14)
  Ground_27/1 -> RFBeacon_3_Tx_Mux/16(spare_15)

== system Demo_TrafficFlow_cs/Mazda_RX8_1 ==
  note: Simple Longitudinal Advanced Cruise Control
      | with the following inputs:
      | - Advised  velocity received via Beacon
      | - Range to vehicle in front detected by AIR sensor
  note: Trajectories:
      | - Following path with ACC Velocity
      | - Looping trajectory (Reinsert vehicle on
      |   LANE 1 when triggered by Traffic Controller)
      | - Lane change is randomly executed
      |   when LANE 2 is open for traffic of LANE 1
      |   (misuse of Beacon, as visual interpretation
      |   of matrix sign is not possible)
  SELF_Mazda_RX8_1 : Inport  Tag=SELFInport
  RFOBU_4_Rx : Inport  Tag=OBURxInport  Port=2
  AIR_4 : Inport  Tag=AIRInport  Port=3  OutDataTypeStr=Bus: PRESCAN_AIRSENSORMESSAGE
  AIR_4_Demux : Subsystem  Tag=DemuxAIR  mask(PreScan Demux Block: AIR){DetectionType=BBox, detectableObjects=10}
      mask prompts: DetectionType "Detection Type"; detectableObjects "Max. detectable objects"
      mask help:
      | The Actor Information Receiver sensor provides a fast, simple and generic detection solution which is not tied up to a specific technology and can be used for scenarios in which a simple sensor is needed.
      |
      | For more information, please click Help.
      mask init: same as Demo_TrafficFlow_cs/Audi_A8_1/AIR_1_Demux
      settings: DeleteFcn=PreScanBlockDeleteFcn;
      mask Help: eval('openHelp(411)')
      mask StyleString: popup(Center|BBox|CoG),edit
      mask Display:
      | disp(char('Det. type:         ',['{\it',DetectionTypeStr,'}']),'texmode','on')
      | port_label('output',1,'Range [m]')
      | port_label('output',2,'{\theta} [deg]','texmode','on')
      | port_label('output',3,'{\phi} [deg]','texmode','on')
      | port_label('output',4,'ID [-]','texmode','on')
      | port_label('output',5,'Velocity [m/s]','texmode','on')
      | port_label('output',6,'Heading [deg]','texmode','on')
  Ground_{1..16} (16 alike) : Ground
  Memory : Memory
  Memory1 : Memory
  Memory2 : Memory
  RFOBU_4_Rx_Demux : Subsystem  Tag=DemuxOBURx  mask(PreScan Demux Block:){Technology='RF'}
      mask init:
      | IconTxt = char('','','','Technology:            ',['{\it',Technology,'}']);
      settings: DeleteFcn=PreScanBlockDeleteFcn;
      mask Display:
      | disp(IconTxt,'texmode','on')
      | port_label('output', 1, 'Beacon ID [-]')
      | port_label('output', 2, 'Beacon GPS Lat [deg]')
      | port_label('output', 3, 'Beacon GPS Lon [deg]')
      | port_label('output', 4, 'Beacon GPS Alt [m]')
      | port_label('output', 5, 'BeaconType [-]')
      | port_label('output', 6, 'Advised_Velocity')
      | port_label('output', 7, 'CarID_Lane1')
      | port_label('output', 8, 'CarID_Lane3')
      | port_label('output', 9, 'State_Lane2')
      | port_label('output', 10, 'spare_5')
      | port_label('output', 11, 'spare_6')
      | port_label('output', 12, 'spare_7')
      | port_label('output', 13, 'spare_8')
      | port_label('output', 14, 'spare_9')
      | port_label('output', 15, 'spare_10')
      | port_label('output', 16, 'spare_11')
      | port_label('output', 17, 'spare_12')
      | port_label('output', 18, 'spare_13')
      | port_label('output', 19, 'spare_14')
      | port_label('output', 20, 'spare_15')
  RFOBU_4_Tx_Mux : Subsystem  Tag=MuxOBUTx  mask(PreScan Mux Block:){Technology='RF'}
      mask init:
      | IconTxt = char('','','              Technology:',['{\it                ',Technology,'}']);
      settings: DeleteFcn=PreScanBlockDeleteFcn;
      mask Display:
      | disp(IconTxt,'texmode','on')
      | port_label('input', 1, 'Vehicle Type [-]')
      | port_label('input', 2, 'spare_1')
      | port_label('input', 3, 'spare_2')
      | port_label('input', 4, 'spare_3')
      | port_label('input', 5, 'spare_4')
      | port_label('input', 6, 'spare_5')
      | port_label('input', 7, 'spare_6')
      | port_label('input', 8, 'spare_7')
      | port_label('input', 9, 'spare_8')
      | port_label('input', 10, 'spare_9')
      | port_label('input', 11, 'spare_10')
      | port_label('input', 12, 'spare_11')
      | port_label('input', 13, 'spare_12')
      | port_label('input', 14, 'spare_13')
      | port_label('input', 15, 'spare_14')
      | port_label('input', 16, 'spare_15')
  SELF_Demux : Subsystem  Tag=DemuxSELF
      settings: DeleteFcn=PreScanBlockDeleteFcn;
      mask Type: PreScan Demux Block:
      mask Display:
      | port_label('output',1,'x [m]')
      | port_label('output',2,'y [m]')
      | port_label('output',3,'z [m]','texmode','on')
      | port_label('output',4,'Rotx [deg]','texmode','on')
      | port_label('output',5,'Roty [deg]','texmode','on')
      | port_label('output',6,'Rotz [deg]','texmode','on')
      | port_label('output',7,'GPS lat [deg]','texmode','on')
      | port_label('output',8,'GPS long [deg]','texmode','on')
      | port_label('output',9,'GPS alt [m]','texmode','on')
      | port_label('output',10,'Velocity [m/s]')
      | port_label('output',11,'Heading [deg]')
      | port_label('output',12,'Yaw rate [deg/s]')
  Selector1 : Selector  InputPortWidth=2  IndexOptions=Index vector (dialog)  Indices=[1]  OutputSizes=1
  Selector2 : Selector  InputPortWidth=2  IndexOptions=Index vector (dialog)  Indices=[1]  OutputSizes=1
  Selector3 : Selector  InputPortWidth=2  IndexOptions=Index vector (dialog)  Indices=[1]  OutputSizes=1
  Selector4 : Selector  InputPortWidth=10  IndexOptions=Index vector (dialog)  Indices=[1]  OutputSizes=1
  Selector5 : Selector  InputPortWidth=10  IndexOptions=Index vector (dialog)  Indices=[1]  OutputSizes=1
  Simple_Longitudinal_ACC : Subsystem
  Terminator_{1..33} (33 alike) : Terminator
  Trajectories : Subsystem  Tag=Trajectories
      settings: DeleteFcn=PreScanBlockDeleteFcn;
      mask Display: image(imread('Trajectory.png'));
  STATE_Mazda_RX8_1 : Outport  Tag=StateOutport
  RFOBU_4_Tx : Outport  Tag=AntennaTOutport  Port=2
  wires:
  Selector1/1 -> Memory/1
  Selector2/1 -> Memory2/1
  Selector4/1 -> Simple_Longitudinal_ACC/2(Range [m])
  Selector3/1 -> Memory1/1
  Memory2/1 -> Simple_Longitudinal_ACC/1(Advised Velocity [km/h])
  Selector5/1 -> Simple_Longitudinal_ACC/3(Velocity [m/s])
  Memory/1 -> [state_lane1]
  Memory1/1 -> [state_lane2]
  Simple_Longitudinal_ACC/1(ACC_Velocity [m/s]) -> [ACC_velocity]
  SELF_Mazda_RX8_1/1 -> SELF_Demux/1(SELF)
  RFOBU_4_Rx/1 -> RFOBU_4_Rx_Demux/1(Beacon_Rx)
  AIR_4/1 -> AIR_4_Demux/1(AIR)
  RFOBU_4_Tx_Mux/1(BeaconTx) -> RFOBU_4_Tx/1
  Trajectories/1(State) -> STATE_Mazda_RX8_1/1
  [state_lane2] -> Trajectories/3(StateLANE2 [-])
  [state_lane1] -> Trajectories/2(OBUID_Reinsert [-])
  [ACC_velocity] -> Trajectories/1(Velocity)
  AIR_4_Demux/1(Range [m]) -> Selector4/1
  RFOBU_4_Rx_Demux/6(spare_1) -> Selector2/1
  RFOBU_4_Rx_Demux/7(spare_2) -> Selector1/1
  RFOBU_4_Rx_Demux/9(spare_4) -> Selector3/1
  AIR_4_Demux/5(Velocity [m/s]) -> Selector5/1
  AIR_4_Demux/2(Azimuth [deg]) -> Terminator_1/1
  AIR_4_Demux/3(Elevation [deg]) -> Terminator_2/1
  AIR_4_Demux/4(ID [-]) -> Terminator_3/1
  AIR_4_Demux/6(Heading [deg]) -> Terminator_4/1
  RFOBU_4_Rx_Demux/1(Beacon_ID) -> Terminator_5/1
  RFOBU_4_Rx_Demux/2(GPS_pos_lat_[deg]) -> Terminator_6/1
  RFOBU_4_Rx_Demux/3(GPS_pos_long_[deg]) -> Terminator_7/1
  RFOBU_4_Rx_Demux/4(GPS_pos_alt_[m]) -> Terminator_8/1
  RFOBU_4_Rx_Demux/5(BeaconType [-]) -> Terminator_9/1
  RFOBU_4_Rx_Demux/8(spare_3) -> Terminator_10/1
  RFOBU_4_Rx_Demux/10(spare_5) -> Terminator_11/1
  RFOBU_4_Rx_Demux/11(spare_6) -> Terminator_12/1
  RFOBU_4_Rx_Demux/12(spare_7) -> Terminator_13/1
  RFOBU_4_Rx_Demux/13(spare_8) -> Terminator_14/1
  RFOBU_4_Rx_Demux/14(spare_9) -> Terminator_15/1
  RFOBU_4_Rx_Demux/15(spare_10) -> Terminator_16/1
  RFOBU_4_Rx_Demux/16(spare_11) -> Terminator_17/1
  RFOBU_4_Rx_Demux/17(spare_12) -> Terminator_18/1
  RFOBU_4_Rx_Demux/18(spare_13) -> Terminator_19/1
  RFOBU_4_Rx_Demux/19(spare_14) -> Terminator_20/1
  RFOBU_4_Rx_Demux/20(spare_15) -> Terminator_21/1
  Ground_1/1 -> RFOBU_4_Tx_Mux/1(Vehicle_Type)
  Ground_2/1 -> RFOBU_4_Tx_Mux/2(spare_1)
  Ground_3/1 -> RFOBU_4_Tx_Mux/3(spare_2)
  Ground_4/1 -> RFOBU_4_Tx_Mux/4(spare_3)
  Ground_5/1 -> RFOBU_4_Tx_Mux/5(spare_4)
  Ground_6/1 -> RFOBU_4_Tx_Mux/6(spare_5)
  Ground_7/1 -> RFOBU_4_Tx_Mux/7(spare_6)
  Ground_8/1 -> RFOBU_4_Tx_Mux/8(spare_7)
  Ground_9/1 -> RFOBU_4_Tx_Mux/9(spare_8)
  Ground_10/1 -> RFOBU_4_Tx_Mux/10(spare_9)
  Ground_11/1 -> RFOBU_4_Tx_Mux/11(spare_10)
  Ground_12/1 -> RFOBU_4_Tx_Mux/12(spare_11)
  Ground_13/1 -> RFOBU_4_Tx_Mux/13(spare_12)
  Ground_14/1 -> RFOBU_4_Tx_Mux/14(spare_13)
  Ground_15/1 -> RFOBU_4_Tx_Mux/15(spare_14)
  Ground_16/1 -> RFOBU_4_Tx_Mux/16(spare_15)
  SELF_Demux/1(x [m]) -> Terminator_22/1
  SELF_Demux/2(y [m]) -> Terminator_23/1
  SELF_Demux/3(z [m]) -> Terminator_24/1
  SELF_Demux/4(rotx [deg]) -> Terminator_25/1
  SELF_Demux/5(roty [deg]) -> Terminator_26/1
  SELF_Demux/6(rotz [deg]) -> Terminator_27/1
  SELF_Demux/7(GPS lat [deg]) -> Terminator_28/1
  SELF_Demux/8(GPS long [deg]) -> Terminator_29/1
  SELF_Demux/9(GPS alt [m]) -> Terminator_30/1
  SELF_Demux/10(velocity [m/s]) -> Terminator_31/1
  SELF_Demux/11(heading [deg]) -> Terminator_32/1
  SELF_Demux/12(yaw rate [deg/s]) -> Terminator_33/1

== system Demo_TrafficFlow_cs/Motorcyclist_1 ==
  note: Simple Longitudinal Advanced Cruise Control
      | with the following inputs:
      | - Advised  velocity received via Beacon
      | - Range to vehicle in front detected by AIR sensor
  note: Trajectories:
      | - Following path with ACC Velocity
      | - Looping trajectory (Reinsert vehicle on
      |   LANE 1 when triggered by Traffic Controller)
      | - Lane change is randomly executed
      |   when LANE 2 is open for traffic of LANE 1
      |   (misuse of Beacon, as visual interpretation
      |   of matrix sign is not possible)
  SELF_Motorcyclist_1 : Inport  Tag=SELFInport
  RFOBU_5_Rx : Inport  Tag=OBURxInport  Port=2
  AIR_5 : Inport  Tag=AIRInport  Port=3  OutDataTypeStr=Bus: PRESCAN_AIRSENSORMESSAGE
  AIR_5_Demux : Subsystem  Tag=DemuxAIR  mask(PreScan Demux Block: AIR){DetectionType=BBox, detectableObjects=10}
      mask prompts: DetectionType "Detection Type"; detectableObjects "Max. detectable objects"
      mask help:
      | The Actor Information Receiver sensor provides a fast, simple and generic detection solution which is not tied up to a specific technology and can be used for scenarios in which a simple sensor is needed.
      |
      | For more information, please click Help.
      mask init: same as Demo_TrafficFlow_cs/Audi_A8_1/AIR_1_Demux
      settings: DeleteFcn=PreScanBlockDeleteFcn;
      mask Help: eval('openHelp(411)')
      mask StyleString: popup(Center|BBox|CoG),edit
      mask Display:
      | disp(char('Det. type:         ',['{\it',DetectionTypeStr,'}']),'texmode','on')
      | port_label('output',1,'Range [m]')
      | port_label('output',2,'{\theta} [deg]','texmode','on')
      | port_label('output',3,'{\phi} [deg]','texmode','on')
      | port_label('output',4,'ID [-]','texmode','on')
      | port_label('output',5,'Velocity [m/s]','texmode','on')
      | port_label('output',6,'Heading [deg]','texmode','on')
  Ground_{1..16} (16 alike) : Ground
  Memory : Memory
  Memory1 : Memory
  Memory2 : Memory
  RFOBU_5_Rx_Demux : Subsystem  Tag=DemuxOBURx  mask(PreScan Demux Block:){Technology='RF'}
      mask init:
      | IconTxt = char('','','','Technology:            ',['{\it',Technology,'}']);
      settings: DeleteFcn=PreScanBlockDeleteFcn;
      mask Display:
      | disp(IconTxt,'texmode','on')
      | port_label('output', 1, 'Beacon ID [-]')
      | port_label('output', 2, 'Beacon GPS Lat [deg]')
      | port_label('output', 3, 'Beacon GPS Lon [deg]')
      | port_label('output', 4, 'Beacon GPS Alt [m]')
      | port_label('output', 5, 'BeaconType [-]')
      | port_label('output', 6, 'Advised_Velocity')
      | port_label('output', 7, 'CarID_Lane1')
      | port_label('output', 8, 'CarID_Lane3')
      | port_label('output', 9, 'State_Lane2')
      | port_label('output', 10, 'spare_5')
      | port_label('output', 11, 'spare_6')
      | port_label('output', 12, 'spare_7')
      | port_label('output', 13, 'spare_8')
      | port_label('output', 14, 'spare_9')
      | port_label('output', 15, 'spare_10')
      | port_label('output', 16, 'spare_11')
      | port_label('output', 17, 'spare_12')
      | port_label('output', 18, 'spare_13')
      | port_label('output', 19, 'spare_14')
      | port_label('output', 20, 'spare_15')
  RFOBU_5_Tx_Mux : Subsystem  Tag=MuxOBUTx  mask(PreScan Mux Block:){Technology='RF'}
      mask init:
      | IconTxt = char('','','              Technology:',['{\it                ',Technology,'}']);
      settings: DeleteFcn=PreScanBlockDeleteFcn;
      mask Display:
      | disp(IconTxt,'texmode','on')
      | port_label('input', 1, 'Vehicle Type [-]')
      | port_label('input', 2, 'spare_1')
      | port_label('input', 3, 'spare_2')
      | port_label('input', 4, 'spare_3')
      | port_label('input', 5, 'spare_4')
      | port_label('input', 6, 'spare_5')
      | port_label('input', 7, 'spare_6')
      | port_label('input', 8, 'spare_7')
      | port_label('input', 9, 'spare_8')
      | port_label('input', 10, 'spare_9')
      | port_label('input', 11, 'spare_10')
      | port_label('input', 12, 'spare_11')
      | port_label('input', 13, 'spare_12')
      | port_label('input', 14, 'spare_13')
      | port_label('input', 15, 'spare_14')
      | port_label('input', 16, 'spare_15')
  SELF_Demux : Subsystem  Tag=DemuxSELF
      settings: DeleteFcn=PreScanBlockDeleteFcn;
      mask Type: PreScan Demux Block:
      mask Display:
      | port_label('output',1,'x [m]')
      | port_label('output',2,'y [m]')
      | port_label('output',3,'z [m]','texmode','on')
      | port_label('output',4,'Rotx [deg]','texmode','on')
      | port_label('output',5,'Roty [deg]','texmode','on')
      | port_label('output',6,'Rotz [deg]','texmode','on')
      | port_label('output',7,'GPS lat [deg]','texmode','on')
      | port_label('output',8,'GPS long [deg]','texmode','on')
      | port_label('output',9,'GPS alt [m]','texmode','on')
      | port_label('output',10,'Velocity [m/s]')
      | port_label('output',11,'Heading [deg]')
      | port_label('output',12,'Yaw rate [deg/s]')
  Selector1 : Selector  InputPortWidth=2  IndexOptions=Index vector (dialog)  Indices=[1]  OutputSizes=1
  Selector2 : Selector  InputPortWidth=2  IndexOptions=Index vector (dialog)  Indices=[1]  OutputSizes=1
  Selector3 : Selector  InputPortWidth=2  IndexOptions=Index vector (dialog)  Indices=[1]  OutputSizes=1
  Selector4 : Selector  InputPortWidth=10  IndexOptions=Index vector (dialog)  Indices=[1]  OutputSizes=1
  Selector5 : Selector  InputPortWidth=10  IndexOptions=Index vector (dialog)  Indices=[1]  OutputSizes=1
  Simple_Longitudinal_ACC : Subsystem
  Terminator_{1..33} (33 alike) : Terminator
  Trajectories : Subsystem  Tag=Trajectories
      settings: DeleteFcn=PreScanBlockDeleteFcn;
      mask Display: image(imread('Trajectory.png'));
  STATE_Motorcyclist_1 : Outport  Tag=StateOutport
  RFOBU_5_Tx : Outport  Tag=AntennaTOutport  Port=2
  wires:
  Selector1/1 -> Memory/1
  Selector2/1 -> Memory2/1
  Selector3/1 -> Memory1/1
  Memory2/1 -> Simple_Longitudinal_ACC/1(Advised Velocity [km/h])
  Selector4/1 -> Simple_Longitudinal_ACC/2(Range [m])
  Selector5/1 -> Simple_Longitudinal_ACC/3(Velocity [m/s])
  Memory/1 -> [state_lane1]
  Memory1/1 -> [state_lane2]
  Simple_Longitudinal_ACC/1(ACC_Velocity [m/s]) -> [ACC_velocity]
  SELF_Motorcyclist_1/1 -> SELF_Demux/1(SELF)
  RFOBU_5_Rx/1 -> RFOBU_5_Rx_Demux/1(Beacon_Rx)
  AIR_5/1 -> AIR_5_Demux/1(AIR)
  RFOBU_5_Tx_Mux/1(BeaconTx) -> RFOBU_5_Tx/1
  Trajectories/1(State) -> STATE_Motorcyclist_1/1
  [state_lane2] -> Trajectories/3(StateLANE2 [-])
  [state_lane1] -> Trajectories/2(OBUID_Reinsert [-])
  [ACC_velocity] -> Trajectories/1(Velocity)
  AIR_5_Demux/1(Range [m]) -> Selector4/1
  RFOBU_5_Rx_Demux/6(spare_1) -> Selector2/1
  RFOBU_5_Rx_Demux/7(spare_2) -> Selector1/1
  RFOBU_5_Rx_Demux/9(spare_4) -> Selector3/1
  AIR_5_Demux/5(Velocity [m/s]) -> Selector5/1
  AIR_5_Demux/2(Azimuth [deg]) -> Terminator_1/1
  AIR_5_Demux/3(Elevation [deg]) -> Terminator_2/1
  AIR_5_Demux/4(ID [-]) -> Terminator_3/1
  AIR_5_Demux/6(Heading [deg]) -> Terminator_4/1
  RFOBU_5_Rx_Demux/1(Beacon_ID) -> Terminator_5/1
  RFOBU_5_Rx_Demux/2(GPS_pos_lat_[deg]) -> Terminator_6/1
  RFOBU_5_Rx_Demux/3(GPS_pos_long_[deg]) -> Terminator_7/1
  RFOBU_5_Rx_Demux/4(GPS_pos_alt_[m]) -> Terminator_8/1
  RFOBU_5_Rx_Demux/5(BeaconType [-]) -> Terminator_9/1
  RFOBU_5_Rx_Demux/8(spare_3) -> Terminator_10/1
  RFOBU_5_Rx_Demux/10(spare_5) -> Terminator_11/1
  RFOBU_5_Rx_Demux/11(spare_6) -> Terminator_12/1
  RFOBU_5_Rx_Demux/12(spare_7) -> Terminator_13/1
  RFOBU_5_Rx_Demux/13(spare_8) -> Terminator_14/1
  RFOBU_5_Rx_Demux/14(spare_9) -> Terminator_15/1
  RFOBU_5_Rx_Demux/15(spare_10) -> Terminator_16/1
  RFOBU_5_Rx_Demux/16(spare_11) -> Terminator_17/1
  RFOBU_5_Rx_Demux/17(spare_12) -> Terminator_18/1
  RFOBU_5_Rx_Demux/18(spare_13) -> Terminator_19/1
  RFOBU_5_Rx_Demux/19(spare_14) -> Terminator_20/1
  RFOBU_5_Rx_Demux/20(spare_15) -> Terminator_21/1
  Ground_1/1 -> RFOBU_5_Tx_Mux/1(Vehicle_Type)
  Ground_2/1 -> RFOBU_5_Tx_Mux/2(spare_1)
  Ground_3/1 -> RFOBU_5_Tx_Mux/3(spare_2)
  Ground_4/1 -> RFOBU_5_Tx_Mux/4(spare_3)
  Ground_5/1 -> RFOBU_5_Tx_Mux/5(spare_4)
  Ground_6/1 -> RFOBU_5_Tx_Mux/6(spare_5)
  Ground_7/1 -> RFOBU_5_Tx_Mux/7(spare_6)
  Ground_8/1 -> RFOBU_5_Tx_Mux/8(spare_7)
  Ground_9/1 -> RFOBU_5_Tx_Mux/9(spare_8)
  Ground_10/1 -> RFOBU_5_Tx_Mux/10(spare_9)
  Ground_11/1 -> RFOBU_5_Tx_Mux/11(spare_10)
  Ground_12/1 -> RFOBU_5_Tx_Mux/12(spare_11)
  Ground_13/1 -> RFOBU_5_Tx_Mux/13(spare_12)
  Ground_14/1 -> RFOBU_5_Tx_Mux/14(spare_13)
  Ground_15/1 -> RFOBU_5_Tx_Mux/15(spare_14)
  Ground_16/1 -> RFOBU_5_Tx_Mux/16(spare_15)
  SELF_Demux/1(x [m]) -> Terminator_22/1
  SELF_Demux/2(y [m]) -> Terminator_23/1
  SELF_Demux/3(z [m]) -> Terminator_24/1
  SELF_Demux/4(rotx [deg]) -> Terminator_25/1
  SELF_Demux/5(roty [deg]) -> Terminator_26/1
  SELF_Demux/6(rotz [deg]) -> Terminator_27/1
  SELF_Demux/7(GPS lat [deg]) -> Terminator_28/1
  SELF_Demux/8(GPS long [deg]) -> Terminator_29/1
  SELF_Demux/9(GPS alt [m]) -> Terminator_30/1
  SELF_Demux/10(velocity [m/s]) -> Terminator_31/1
  SELF_Demux/11(heading [deg]) -> Terminator_32/1
  SELF_Demux/12(yaw rate [deg/s]) -> Terminator_33/1
